simulink model "TosAeb"  (R2021a)
settings: Solver=FixedStepDiscrete  StartTime=0.0  StopTime=inf  FixedStep=0.01  RelTol=1e-3
undefined symbols (not defined in the file or in its folder): ACCModSts, AccSts1, AEB_ObjectMovingOrientation, AEB_ObjectMovingState, CN_BTCActive, CN_CurrentGearR, CN_CurrentGearR_DHT, CN_PTCActive, CN_VDCActive, CN_VSC_EspOff, CN_VSC_FCW_AEB_Error, CN_VSC_FCW_AEB_NoDispay, CN_VSC_FCW_AEB_PerfmDgdtn, CN_VSC_FCW_AEB_TempUnAvlbl, CN_VSC_VehStandstill, CNaccelAvoidMax, CNaccelAvoidMin, CNbrakePedalPress, CNcomfortStrLeftAccelMax, CNcomfortStrLeftAccelMin, CNcomfortStrRightAccelMax, CNcomfortStrRightAccelMin, CNDrvDoorOpen, CNDrvModeEco, CNDrvModeNorm, CNDrvModeOther, CNDrvModeSport, CNDrvSBRreminder, CNNull, CNobjInvalidID, CNPassDoorOpen, CNPassSBRreminder, CNPEBSAccelNegInf, CNPEBSAccelNull, CNPEBSTimeInf, CNPEBSTimeNull, CNSTOPMAX, CNSTOPNULL, CNsubjWidth, CNSwtOFF, CNSwtON, CNTTCMAX, CNTTCNULL, CNVehConfigAEBCycNone, CNVehConfigAEBNone, CNVehConfigAEBPedNone, CNVehConfigFCWNone, CNVehConfigJANone, CollisionSeverity, FALSE, FCWSnvty, JACycObjCalcInfoDefaultParams, JAPedObjCalcInfoDefaultParams, JAVehObjCalcInfoDefaultParams, latCycObjCalcInfoDefaultParams, latPedObjCalcInfoDefaultParams, longObjCalcInfoDefaultParams, ModSts, movingStatus, ObjectType ... (+1112)
libraries: simulink x3031, AEBSFunctionLib x50, Ta_Library x33, AEBSStateMachineLib x23, Mylibrary x12, simulink_extras x7, ObjSelect_lib x3, dspstat3 x2
(part 52 of 102)

== system TosAeb/FcwAebJa_Step_sys/ALG_AEBSystem/ALG_AEBController/AEB_FuncCondCalcApp/JaPedFunctionCondCalc/AEBJAPedPrefillSuppAborCond/AEBJAPedPrefillSuppCondCalc/JaPedPrefillSuppSubjCondCalcu/brakeReleventSuppCond ==
  VehConfigIbooster : Inport
  BrakePedalStatus_G : Inport  Port=2
  ESP_MasterCylindBrakePress : Inport  Port=3
  IB_BrkPedalAppld : Inport  Port=4
  Compare To Constant : simulink/Logic and Bit Operations/Compare To Constant  relop===  const=FALSE  OutDataTypeStr=boolean
  If : If  IfExpression=u1
  Merge : Merge
  brakeReleventSuppCond_ESP : Subsystem  VariantControl=(default)
  brakeReleventSuppCond_IBooster : Subsystem  VariantControl=P_AEB_PowerTrainConfig == 1
  brakeReleventSuppCond : Outport
  wires:
  BrakePedalStatus_G/1 -> brakeReleventSuppCond_ESP/1(BrakePedalStatus_G)
  ESP_MasterCylindBrakePress/1 -> brakeReleventSuppCond_ESP/2(ESP_MasterCylindBrakePress)
  IB_BrkPedalAppld/1 -> brakeReleventSuppCond_IBooster/1(IB_BrkPedalAppld)
  Merge/1 -> brakeReleventSuppCond/1
  brakeReleventSuppCond_IBooster/1(brakeReleventSuppCond) -> Merge/2
  brakeReleventSuppCond_ESP/1(brakeReleventSuppCond) -> Merge/1
  If/2 -> brakeReleventSuppCond_IBooster/Action
  If/1 -> brakeReleventSuppCond_ESP/Action
  Compare To Constant/1 -> If/1
  VehConfigIbooster/1 -> Compare To Constant/1

== system TosAeb/FcwAebJa_Step_sys/ALG_AEBSystem/ALG_AEBController/AEB_FuncCondCalcApp/JaVehFunctionCondCalc/AEBJAVehAEBfullSuppAborCond/AEBJAVehAEBfullSuppCondCalc/AEBJAVehAEBfullSuppSubjCondCalcu/brakeReleventSuppCond ==
  VehConfigIbooster : Inport
  ESP_MasterCylindBrakePress : Inport  Port=2
  Compare To Constant : simulink/Logic and Bit Operations/Compare To Constant  relop===  const=FALSE  OutDataTypeStr=boolean
  If : If  IfExpression=u1
  Merge : Merge
  brakeReleventSuppCond_ESP : Subsystem  VariantControl=(default)
  brakeReleventSuppCond_IBooster : Subsystem  VariantControl=P_AEB_PowerTrainConfig == 1
  brakeReleventSuppCond : Outport
  wires:
  ESP_MasterCylindBrakePress/1 -> brakeReleventSuppCond_ESP/1(ESP_MasterCylindBrakePress)
  Merge/1 -> brakeReleventSuppCond/1
  brakeReleventSuppCond_IBooster/1(brakeReleventSuppCond) -> Merge/2
  brakeReleventSuppCond_ESP/1(brakeReleventSuppCond) -> Merge/1
  If/2 -> brakeReleventSuppCond_IBooster/Action
  If/1 -> brakeReleventSuppCond_ESP/Action
  Compare To Constant/1 -> If/1
  VehConfigIbooster/1 -> Compare To Constant/1

== system TosAeb/FcwAebJa_Step_sys/ALG_AEBSystem/ALG_AEBController/AEB_FuncCondCalcApp/JaVehFunctionCondCalc/AEBJAVehPrefillSuppAborCond/AEBJAVehPrefillSuppCondCalc/AEBJAVehPrefillSuppSubjCondCalcu/brakeReleventSuppCond ==
  VehConfigIbooster : Inport
  BrakePedalStatus_G : Inport  Port=2
  ESP_MasterCylindBrakePress : Inport  Port=3
  IB_BrkPedalAppld : Inport  Port=4
  Compare To Constant : simulink/Logic and Bit Operations/Compare To Constant  relop===  const=FALSE  OutDataTypeStr=boolean
  If : If  IfExpression=u1
  Merge : Merge
  brakeReleventSuppCond_ESP : Subsystem  VariantControl=(default)
  brakeReleventSuppCond_IBooster : Subsystem  VariantControl=P_AEB_PowerTrainConfig == 1
  brakeReleventSuppCond : Outport
  wires:
  ESP_MasterCylindBrakePress/1 -> brakeReleventSuppCond_ESP/2(ESP_MasterCylindBrakePress)
  BrakePedalStatus_G/1 -> brakeReleventSuppCond_ESP/1(BrakePedalStatus_G)
  IB_BrkPedalAppld/1 -> brakeReleventSuppCond_IBooster/1(IB_BrkPedalAppld)
  Merge/1 -> brakeReleventSuppCond/1
  brakeReleventSuppCond_IBooster/1(brakeReleventSuppCond) -> Merge/2
  brakeReleventSuppCond_ESP/1(brakeReleventSuppCond) -> Merge/1
  If/2 -> brakeReleventSuppCond_IBooster/Action
  If/1 -> brakeReleventSuppCond_ESP/Action
  Compare To Constant/1 -> If/1
  VehConfigIbooster/1 -> Compare To Constant/1

== system TosAeb/FcwAebJa_Step_sys/ALG_AEBSystem/ALG_AEBController/AEB_FuncCondCalcApp/LongFunctionCondCalc/vehABASuppAborCondCalc/vehABAAborCondCalc/vehABAVehRelAborCondCalc/DriverAborCondJudge ==
  SteerWheelAngle : Inport
  SteerWheelSpd : Inport  Port=2
  AccrPedlPosnDiagc : Inport  Port=3
  Abs : Abs
  Abs1 : Abs
  Compare To Constant : simulink/Logic and Bit Operations/Compare To Constant  relop=>  const=P_AEB_Func_vehABAStrWheelAngleAborThres  OutDataTypeStr=boolean
  Compare To Constant1 : simulink/Logic and Bit Operations/Compare To Constant  relop=>  const=P_AEB_Func_vehABAStrWheelAngleSpdAborThres  OutDataTypeStr=boolean
  Compare To Constant4 : simulink/Logic and Bit Operations/Compare To Constant  relop=>  const=P_AEB_Func_vehABAGasPedalAborThres  OutDataTypeStr=boolean
  Logical Operator : Logic  Operator=OR  AllPortsSameDT=off  OutDataTypeStr=boolean
  Logical Operator2 : Logic  Operator=OR  AllPortsSameDT=off  OutDataTypeStr=boolean
  driverAborCondFlg : Outport
  wires:
  Logical Operator2/1 -> driverAborCondFlg/1  "driverAborCond"
  Compare To Constant4/1 -> Logical Operator2/2  "accrlReleventAborCond"
  AccrPedlPosnDiagc/1 -> Compare To Constant4/1
  Logical Operator/1 -> Logical Operator2/1  "strReleventAborCond"
  Compare To Constant1/1 -> Logical Operator/2
  Compare To Constant/1 -> Logical Operator/1
  Abs1/1 -> Compare To Constant1/1
  SteerWheelSpd/1 -> Abs1/1
  Abs/1 -> Compare To Constant/1
  SteerWheelAngle/1 -> Abs/1

== system TosAeb/FcwAebJa_Step_sys/ALG_AEBSystem/ALG_AEBController/AEB_FuncCondCalcApp/LongFunctionCondCalc/vehABASuppAborCondCalc/vehABAAborCondCalc/vehABAVehRelAborCondCalc/vehPreWarningVehRelActiveCondJudge ==
  driverAborCondFlg : Inport
  vehStateCalcABAAborFlg : Inport  Port=2
  Compare To Constant : simulink/Logic and Bit Operations/Compare To Constant  relop===  const=TRUE  OutDataTypeStr=boolean
  Compare To Constant1 : simulink/Logic and Bit Operations/Compare To Constant  relop===  const=TRUE  OutDataTypeStr=boolean
  Logical Operator1 : Logic  Operator=OR  AllPortsSameDT=off  OutDataTypeStr=boolean
  vehABAVehRelAborCondFulfilled : Outport
  wires:
  Logical Operator1/1 -> vehABAVehRelAborCondFulfilled/1
  vehStateCalcABAAborFlg/1 -> Compare To Constant1/1
  driverAborCondFlg/1 -> Compare To Constant/1
  Compare To Constant/1 -> Logical Operator1/1
  Compare To Constant1/1 -> Logical Operator1/2

== system TosAeb/FcwAebJa_Step_sys/ALG_AEBSystem/ALG_AEBController/AEB_FuncCondCalcApp/LongFunctionCondCalc/vehABASuppAborCondCalc/vehABASuppCondCalc/vehABAVehRelActiveCondCalc/DriverCondJudge ==
  SteerWheelAngle : Inport
  SteerWheelSpd : Inport  Port=2
  AccrPedlPosnDiagc : Inport  Port=3
  Abs : Abs
  Abs1 : Abs
  AccrlReleventSuppCondStateDelayAbortion : AEBSFunctionLib/StateDelayAbortion  SystemSampleTime=-1  VariantActivationTime=update diagram  Latency=0  AutoFrameSizeCalculation=off
  Compare To Constant : simulink/Logic and Bit Operations/Compare To Constant  relop=>  const=P_AEB_Func_vehABAStrWheelAngleActivationThres  OutDataTypeStr=boolean
  Compare To Constant1 : simulink/Logic and Bit Operations/Compare To Constant  relop=>  const=P_AEB_Func_vehABAStrWheelAngleSpdActivationThres  OutDataTypeStr=boolean
  Compare To Constant4 : simulink/Logic and Bit Operations/Compare To Constant  relop=>  const=P_AEB_Func_vehABAGasPedalActivationThres  OutDataTypeStr=boolean
  Constant : Constant  Value=P_AEB_Func_vehABAStrCondAborDelayTime
  Constant1 : Constant  Value=Ts
  Constant2 : Constant  Value=P_AEB_Func_vehABAAccrCondAborDelayTime
  Constant3 : Constant  Value=Ts
  Logical Operator : Logic  Operator=OR  AllPortsSameDT=off  OutDataTypeStr=boolean
  Logical Operator2 : Logic  AllPortsSameDT=off  OutDataTypeStr=boolean
  StrReleventSuppCondStateDelayAbortion : AEBSFunctionLib/StateDelayAbortion  SystemSampleTime=-1  VariantActivationTime=update diagram  Latency=0  AutoFrameSizeCalculation=off
  driverSuppCondFlg : Outport
  wires:
  Constant3/1 -> AccrlReleventSuppCondStateDelayAbortion/3
  Constant2/1 -> AccrlReleventSuppCondStateDelayAbortion/2
  Compare To Constant4/1 -> AccrlReleventSuppCondStateDelayAbortion/1
  Constant1/1 -> StrReleventSuppCondStateDelayAbortion/3
  Constant/1 -> StrReleventSuppCondStateDelayAbortion/2
  Logical Operator/1 -> StrReleventSuppCondStateDelayAbortion/1
  SteerWheelAngle/1 -> Abs/1
  Abs/1 -> Compare To Constant/1
  SteerWheelSpd/1 -> Abs1/1
  Abs1/1 -> Compare To Constant1/1
  Compare To Constant/1 -> Logical Operator/1
  Compare To Constant1/1 -> Logical Operator/2
  StrReleventSuppCondStateDelayAbortion/1 -> Logical Operator2/1  "strReleventSuppCond"
  AccrPedlPosnDiagc/1 -> Compare To Constant4/1
  AccrlReleventSuppCondStateDelayAbortion/1 -> Logical Operator2/2  "accrlReleventSuppCond"
  Logical Operator2/1 -> driverSuppCondFlg/1  "driverSuppCond"

== system TosAeb/FcwAebJa_Step_sys/ALG_AEBSystem/ALG_AEBController/AEB_FuncCondCalcApp/LongFunctionCondCalc/vehABASuppAborCondCalc/vehABASuppCondCalc/vehABAVehRelActiveCondCalc/subjVelWork ==
  ObjectMovingState : Inport
  Compare To Constant : simulink/Logic and Bit Operations/Compare To Constant  relop===  const=AEB_ObjectMovingState.Still  OutDataTypeStr=boolean
  Constant : Constant  Value=P_AEB_Func_vehABASubjVelMaxSO
  Constant2 : Constant  Value=P_AEB_Func_vehABASubjVelMin
  Constant3 : Constant  Value=P_AEB_Func_vehABASubjVelMaxMO
  Switch1 : Switch  Criteria=u2 ~= 0
  subjVelWorkMax : Outport
  subjVelWorkMin : Outport  Port=2
  wires:
  Switch1/1 -> subjVelWorkMax/1
  Constant3/1 -> Switch1/3
  Compare To Constant/1 -> Switch1/2
  Constant2/1 -> subjVelWorkMin/1
  ObjectMovingState/1 -> Compare To Constant/1
  Constant/1 -> Switch1/1

== system TosAeb/FcwAebJa_Step_sys/ALG_AEBSystem/ALG_AEBController/AEB_FuncCondCalcApp/LongFunctionCondCalc/vehABASuppAborCondCalc/vehABASuppCondCalc/vehABAVehRelActiveCondCalc/vehABAVehRelActiveCondJudge ==
  subjVel : Inport
  subjVelWorkMax : Inport  Port=2
  subjVelWorkMin : Inport  Port=3
  driverSuppCondFlg : Inport  Port=4
  vehStateCalcABASuppFlg : Inport  Port=5
  Compare To Constant : simulink/Logic and Bit Operations/Compare To Constant  relop===  const=FALSE  OutDataTypeStr=boolean
  Compare To Constant1 : simulink/Logic and Bit Operations/Compare To Constant  relop===  const=FALSE  OutDataTypeStr=boolean
  Logical Operator : Logic  AllPortsSameDT=off  OutDataTypeStr=boolean
  Logical Operator1 : Logic  Inputs=3  AllPortsSameDT=off  OutDataTypeStr=boolean
  Relational Operator : RelationalOperator  Operator=<  OutDataTypeStr=boolean  RndMeth=Simplest
  Relational Operator1 : RelationalOperator  Operator=>  OutDataTypeStr=boolean  RndMeth=Simplest
  vehABAVehRelActiveCondFulfilled : Outport
  wires:
  Compare To Constant1/1 -> Logical Operator1/3
  subjVel/1 -> Relational Operator1/1, Relational Operator/1
  subjVelWorkMax/1 -> Relational Operator/2
  Relational Operator/1 -> Logical Operator/1
  Relational Operator1/1 -> Logical Operator/2
  driverSuppCondFlg/1 -> Compare To Constant/1
  vehStateCalcABASuppFlg/1 -> Compare To Constant1/1
  Compare To Constant/1 -> Logical Operator1/2
  Logical Operator/1 -> Logical Operator1/1
  Logical Operator1/1 -> vehABAVehRelActiveCondFulfilled/1
  subjVelWorkMin/1 -> Relational Operator1/2

== system TosAeb/FcwAebJa_Step_sys/ALG_AEBSystem/ALG_AEBController/AEB_FuncCondCalcApp/LongFunctionCondCalc/vehAEBfullSuppAborCondCalc/ vehAEBfullSuppCondCalc/vehAEBfullSituationRelActiveCondCalc/overlapThesCalc ==
  ObjectTypeCameraConfirmed : Inport
  dxFrontBumper : Inport  Port=2
  Compare To Constant : simulink/Logic and Bit Operations/Compare To Constant  relop===  const=TRUE  OutDataTypeStr=boolean
  If : If  IfExpression=u1
  If Action Subsystem : Subsystem  TreatAsAtomicUnit=on
  If Action Subsystem1 : Subsystem  TreatAsAtomicUnit=on
  Merge : Merge
  overlapThes : Outport
  wires:
  ObjectTypeCameraConfirmed/1 -> Compare To Constant/1
  dxFrontBumper/1 -> If Action Subsystem1/1(dxFrontBumper), If Action Subsystem/1(dxFrontBumper)
  If Action Subsystem/1(overlapThes) -> Merge/1
  Compare To Constant/1 -> If/1
  [ObjectNoFusion] -> If Action Subsystem1/Action
  Merge/1 -> overlapThes/1
  If/2 -> [ObjectNoFusion]
  If Action Subsystem1/1(overlapThes) -> Merge/2
  If/1 -> If Action Subsystem/Action

== system TosAeb/FcwAebJa_Step_sys/ALG_AEBSystem/ALG_AEBController/AEB_FuncCondCalcApp/LongFunctionCondCalc/vehAEBfullSuppAborCondCalc/ vehAEBfullSuppCondCalc/vehAEBfullSituationRelActiveCondCalc/vehAEBfullSituationRelActiveCondCalc ==
  overlapThes : Inport
  longCollisionSeverity : Inport  Port=2
  overlapRatio : Inport  Port=3
  LCK_Active : Inport  Port=4
  probEscape : Inport  Port=5
  subjCurvTraj : Inport  Port=6
  Compare To Constant1 : simulink/Logic and Bit Operations/Compare To Constant  relop===  const=TRUE  OutDataTypeStr=boolean
  Compare To Constant2 : simulink/Logic and Bit Operations/Compare To Constant  relop===  const=CollisionSeverity.High  OutDataTypeStr=boolean
  Compare To Constant3 : simulink/Logic and Bit Operations/Compare To Constant  relop=>  const=P_AEB_Func_vehAEBfullEscapeActivationThres  OutDataTypeStr=boolean
  Compare To Constant4 : simulink/Logic and Bit Operations/Compare To Constant  relop=<  const=P_AEB_Func_vehAEBfullSubjCurvTrajActivationThres  OutDataTypeStr=boolean
  Compare To Constant5 : simulink/Logic and Bit Operations/Compare To Constant  relop===  const=FALSE  OutDataTypeStr=boolean
  Constant : Constant  Value=TRUE
  Logical Operator1 : Logic  Inputs=4  AllPortsSameDT=off  OutDataTypeStr=boolean
  Relational Operator : RelationalOperator  Operator=>  OutDataTypeStr=boolean  RndMeth=Simplest
  Switch : Switch  Criteria=u2 ~= 0
  vehAEBfullSituationRelActiveCondFulfilled : Outport
  wires:
  Constant/1 -> Switch/1
  Switch/1 -> Logical Operator1/3
  Compare To Constant1/1 -> Switch/2
  LCK_Active/1 -> Compare To Constant1/1
  longCollisionSeverity/1 -> Compare To Constant2/1
  subjCurvTraj/1 -> Compare To Constant4/1
  overlapThes/1 -> Relational Operator/2
  overlapRatio/1 -> Relational Operator/1
  probEscape/1 -> Compare To Constant3/1
  Compare To Constant3/1 -> Compare To Constant5/1  "probEscapeFulfilled"
  Compare To Constant5/1 -> Switch/3
  Relational Operator/1 -> Logical Operator1/2  "OverlapCondFulfilled"
  Compare To Constant4/1 -> Logical Operator1/4  "latStableCondFulfilled"
  Compare To Constant2/1 -> Logical Operator1/1  "collisionLvlCondFulfilled"
  Logical Operator1/1 -> vehAEBfullSituationRelActiveCondFulfilled/1

== system TosAeb/FcwAebJa_Step_sys/ALG_AEBSystem/ALG_AEBController/AEB_FuncCondCalcApp/LongFunctionCondCalc/vehAEBfullSuppAborCondCalc/ vehAEBfullSuppCondCalc/vehAEBfullVehRelActiveCondCalc/DriverCondJudge ==
  SteerWheelAngle : Inport
  SteerWheelSpd : Inport  Port=2
  VehConfigIbooster : Inport  Port=3
  ESPmasterCylindBrakePress : Inport  Port=4
  BrakePedalStatus_G : Inport  Port=5
  subjLongAccel : Inport  Port=6
  subjVel : Inport  Port=7
  AccrPedlPosnDiagc : Inport  Port=8
  Abs : Abs
  Abs1 : Abs
  Compare To Constant : simulink/Logic and Bit Operations/Compare To Constant  relop=>  const=P_AEB_Func_vehAEBfullStrWheelAngleActivationThres  OutDataTypeStr=boolean
  Compare To Constant1 : simulink/Logic and Bit Operations/Compare To Constant  relop=>  const=P_AEB_Func_vehAEBfullStrWheelAngleSpdActivationThres  OutDataTypeStr=boolean
  Compare To Constant4 : simulink/Logic and Bit Operations/Compare To Constant  relop=>  const=P_AEB_Func_vehAEBfullGasPedalActivationThres  OutDataTypeStr=boolean
  Constant : Constant  Value=P_AEB_Func_vehAEBfullStrCondAborDelayTime
  Constant1 : Constant  Value=Ts
  Constant2 : Constant  Value=P_AEB_Func_vehAEBfullAccrCondAborDelayTime
  Constant3 : Constant  Value=Ts
  Logical Operator : Logic  Operator=OR  AllPortsSameDT=off  OutDataTypeStr=boolean
  Logical Operator2 : Logic  Operator=OR  Inputs=3  AllPortsSameDT=off  OutDataTypeStr=boolean
  accrlReleventSuppCondStateDelayAbortion : AEBSFunctionLib/StateDelayAbortion  SystemSampleTime=-1  VariantActivationTime=update diagram  Latency=0  AutoFrameSizeCalculation=off
  brakeReleventSuppCond : Subsystem
  strReleventSuppCondStateDelayAbortion : AEBSFunctionLib/StateDelayAbortion  SystemSampleTime=-1  VariantActivationTime=update diagram  Latency=0  AutoFrameSizeCalculation=off
  driverSuppCondFlg : Outport
  wires:
  VehConfigIbooster/1 -> brakeReleventSuppCond/1(VehConfigIbooster)
  ESPmasterCylindBrakePress/1 -> brakeReleventSuppCond/2(ESPmasterCylindBrakePress)
  subjVel/1 -> brakeReleventSuppCond/5(subjVel)
  BrakePedalStatus_G/1 -> brakeReleventSuppCond/3(BrakePedalStatus_G)
  subjLongAccel/1 -> brakeReleventSuppCond/4(subjLongAccel)
  Constant3/1 -> accrlReleventSuppCondStateDelayAbortion/3
  Constant2/1 -> accrlReleventSuppCondStateDelayAbortion/2
  Compare To Constant4/1 -> accrlReleventSuppCondStateDelayAbortion/1
  Constant1/1 -> strReleventSuppCondStateDelayAbortion/3
  Constant/1 -> strReleventSuppCondStateDelayAbortion/2
  SteerWheelAngle/1 -> Abs/1
  Abs/1 -> Compare To Constant/1
  SteerWheelSpd/1 -> Abs1/1
  Abs1/1 -> Compare To Constant1/1
  Compare To Constant/1 -> Logical Operator/1
  Compare To Constant1/1 -> Logical Operator/2
  Logical Operator/1 -> strReleventSuppCondStateDelayAbortion/1
  strReleventSuppCondStateDelayAbortion/1 -> Logical Operator2/1  "strReleventSuppCond"
  brakeReleventSuppCond/1(brakeReleventSuppCond) -> Logical Operator2/2  "brakeReleventSuppCond"
  AccrPedlPosnDiagc/1 -> Compare To Constant4/1
  accrlReleventSuppCondStateDelayAbortion/1 -> Logical Operator2/3  "accrlReleventSuppCond"
  Logical Operator2/1 -> driverSuppCondFlg/1  "driverSuppCond"

== system TosAeb/FcwAebJa_Step_sys/ALG_AEBSystem/ALG_AEBController/AEB_FuncCondCalcApp/LongFunctionCondCalc/vehAEBfullSuppAborCondCalc/ vehAEBfullSuppCondCalc/vehAEBfullVehRelActiveCondCalc/subjVelWork ==
  ObjectTypeCameraConfirmed : Inport
  ObjectMovingState : Inport  Port=2
  Compare To Constant : simulink/Logic and Bit Operations/Compare To Constant  relop===  const=AEB_ObjectMovingState.Still  OutDataTypeStr=boolean
  Compare To Constant1 : simulink/Logic and Bit Operations/Compare To Constant  relop===  const=TRUE  OutDataTypeStr=boolean
  Constant : Constant  Value=P_AEB_Func_vehAEBfullFusionSubjVelMaxThresSO
  Constant1 : Constant  Value=P_AEB_Func_vehAEBfullNoFusionSubjVelMaxThresSO
  Constant2 : Constant  Value=P_AEB_Func_vehAEBfullSubjVelMinThres
  Constant3 : Constant  Value=P_AEB_Func_vehAEBfullSubjWorkVelMaxThresMO
  Switch : Switch  Criteria=u2 ~= 0
  Switch1 : Switch  Criteria=u2 ~= 0
  subjVelWorkMax : Outport
  subjVelWorkMin : Outport  Port=2
  wires:
  Switch1/1 -> subjVelWorkMax/1
  Constant3/1 -> Switch1/3
  Switch/1 -> Switch1/1
  Compare To Constant/1 -> Switch1/2
  Compare To Constant1/1 -> Switch/2
  ObjectTypeCameraConfirmed/1 -> Compare To Constant1/1
  Constant2/1 -> subjVelWorkMin/1
  Constant1/1 -> Switch/3
  Constant/1 -> Switch/1
  ObjectMovingState/1 -> Compare To Constant/1

== system TosAeb/FcwAebJa_Step_sys/ALG_AEBSystem/ALG_AEBController/AEB_FuncCondCalcApp/LongFunctionCondCalc/vehAEBfullSuppAborCondCalc/ vehAEBfullSuppCondCalc/vehAEBfullVehRelActiveCondCalc/vehAEBfullVehRelActiveCondJudge ==
  subjVel : Inport
  subjVelWorkMax : Inport  Port=2
  subjVelWorkMin : Inport  Port=3
  driverSuppCondFlg : Inport  Port=4
  vehStateCalcAEBfullSuppFlg : Inport  Port=5
  Compare To Constant : simulink/Logic and Bit Operations/Compare To Constant  relop===  const=FALSE  OutDataTypeStr=boolean
  Compare To Constant1 : simulink/Logic and Bit Operations/Compare To Constant  relop===  const=FALSE  OutDataTypeStr=boolean
  Logical Operator : Logic  AllPortsSameDT=off  OutDataTypeStr=boolean
  Logical Operator1 : Logic  Inputs=3  AllPortsSameDT=off  OutDataTypeStr=boolean
  Relational Operator : RelationalOperator  Operator=<  OutDataTypeStr=boolean  RndMeth=Simplest
  Relational Operator1 : RelationalOperator  Operator=>  OutDataTypeStr=boolean  RndMeth=Simplest
  vehAEBfullVehRelActiveCondFulfilled : Outport
  wires:
  Compare To Constant1/1 -> Logical Operator1/3
  subjVel/1 -> Relational Operator1/1, Relational Operator/1
  subjVelWorkMax/1 -> Relational Operator/2
  Relational Operator/1 -> Logical Operator/1
  Relational Operator1/1 -> Logical Operator/2
  driverSuppCondFlg/1 -> Compare To Constant/1
  vehStateCalcAEBfullSuppFlg/1 -> Compare To Constant1/1
  Compare To Constant/1 -> Logical Operator1/2
  Logical Operator/1 -> Logical Operator1/1
  Logical Operator1/1 -> vehAEBfullVehRelActiveCondFulfilled/1  "vehAEBfullVehRelActiveCondFulfilled"
  subjVelWorkMin/1 -> Relational Operator1/2

== system TosAeb/FcwAebJa_Step_sys/ALG_AEBSystem/ALG_AEBController/AEB_FuncCondCalcApp/LongFunctionCondCalc/vehAEBfullSuppAborCondCalc/vehAEBfullAborCondCalc/vehAEBfullVehRelAborCondCalc/DriverAborCondJudge ==
  SteerWheelAngle : Inport
  SteerWheelSpd : Inport  Port=2
  AccrPedlPosnDiagc : Inport  Port=3
  Abs : Abs
  Abs1 : Abs
  Compare To Constant : simulink/Logic and Bit Operations/Compare To Constant  relop=>  const=P_AEB_Func_vehAEBfullStrWheelAngleAborThres  OutDataTypeStr=boolean
  Compare To Constant1 : simulink/Logic and Bit Operations/Compare To Constant  relop=>  const=P_AEB_Func_vehAEBfullStrWheelAngleSpdAborThres  OutDataTypeStr=boolean
  Compare To Constant4 : simulink/Logic and Bit Operations/Compare To Constant  relop=>  const=P_AEB_Func_vehAEBfullGasPedalAborThres  OutDataTypeStr=boolean
  Logical Operator : Logic  Operator=OR  AllPortsSameDT=off  OutDataTypeStr=boolean
  Logical Operator2 : Logic  Operator=OR  AllPortsSameDT=off  OutDataTypeStr=boolean
  driverAborCondFlg : Outport
  wires:
  Logical Operator2/1 -> driverAborCondFlg/1  "driverAborCond"
  Compare To Constant4/1 -> Logical Operator2/2  "accrlReleventAborCond"
  AccrPedlPosnDiagc/1 -> Compare To Constant4/1
  Logical Operator/1 -> Logical Operator2/1  "strReleventAborCond"
  Compare To Constant1/1 -> Logical Operator/2
  Compare To Constant/1 -> Logical Operator/1
  Abs1/1 -> Compare To Constant1/1
  SteerWheelSpd/1 -> Abs1/1
  Abs/1 -> Compare To Constant/1
  SteerWheelAngle/1 -> Abs/1

== system TosAeb/FcwAebJa_Step_sys/ALG_AEBSystem/ALG_AEBController/AEB_FuncCondCalcApp/LongFunctionCondCalc/vehAEBfullSuppAborCondCalc/vehAEBfullAborCondCalc/vehAEBfullVehRelAborCondCalc/vehAEBfullVehRelAborCondJudge ==
  driverAborCondFlg : Inport
  vehStateCalcAEBfullAborFlg : Inport  Port=2
  Compare To Constant : simulink/Logic and Bit Operations/Compare To Constant  relop===  const=TRUE  OutDataTypeStr=boolean
  Compare To Constant1 : simulink/Logic and Bit Operations/Compare To Constant  relop===  const=TRUE  OutDataTypeStr=boolean
  Logical Operator1 : Logic  Operator=OR  AllPortsSameDT=off  OutDataTypeStr=boolean
  vehAEBfullVehRelAborCondFulfilled : Outport
  wires:
  Compare To Constant1/1 -> Logical Operator1/2
  Logical Operator1/1 -> vehAEBfullVehRelAborCondFulfilled/1  "vehAEBfullVehRelAborCondFulfilled"
  Compare To Constant/1 -> Logical Operator1/1
  vehStateCalcAEBfullAborFlg/1 -> Compare To Constant1/1  "vehStateCalcAEBfullAborFlg"
  driverAborCondFlg/1 -> Compare To Constant/1

== system TosAeb/FcwAebJa_Step_sys/ALG_AEBSystem/ALG_AEBController/AEB_FuncCondCalcApp/LongFunctionCondCalc/vehAEBpartSuppAborCondCalc/vehAEBpartAborCondCalc/vehAEBpartVehRelAborCondCalc/DriverAborCondJudge ==
  SteerWheelAngle : Inport
  SteerWheelSpd : Inport  Port=2
  AccrPedlPosnDiagc : Inport  Port=3
  Abs : Abs
  Abs1 : Abs
  Compare To Constant : simulink/Logic and Bit Operations/Compare To Constant  relop=>  const=P_AEB_Func_vehAEBpartStrWheelAngleSpdAborThres  OutDataTypeStr=boolean
  Compare To Constant1 : simulink/Logic and Bit Operations/Compare To Constant  relop=>  const=P_AEB_Func_vehAEBpartStrWheelAngleAborThres  OutDataTypeStr=boolean
  Compare To Constant4 : simulink/Logic and Bit Operations/Compare To Constant  relop=>  const=P_AEB_Func_vehAEBpartGasPedalAborThres  OutDataTypeStr=boolean
  Logical Operator : Logic  Operator=OR  AllPortsSameDT=off  OutDataTypeStr=boolean
  Logical Operator2 : Logic  Operator=OR  AllPortsSameDT=off  OutDataTypeStr=boolean
  driverAborCondFlg : Outport
  wires:
  SteerWheelAngle/1 -> Abs/1
  Abs/1 -> Compare To Constant/1
  SteerWheelSpd/1 -> Abs1/1
  Abs1/1 -> Compare To Constant1/1
  Compare To Constant/1 -> Logical Operator/1
  Compare To Constant1/1 -> Logical Operator/2
  Logical Operator/1 -> Logical Operator2/1  "strReleventAborCond"
  AccrPedlPosnDiagc/1 -> Compare To Constant4/1
  Compare To Constant4/1 -> Logical Operator2/2  "accrlReleventAborCond"
  Logical Operator2/1 -> driverAborCondFlg/1  "driverAborCondFlg"

== system TosAeb/FcwAebJa_Step_sys/ALG_AEBSystem/ALG_AEBController/AEB_FuncCondCalcApp/LongFunctionCondCalc/vehAEBpartSuppAborCondCalc/vehAEBpartAborCondCalc/vehAEBpartVehRelAborCondCalc/vehPreWarningVehRelActiveCondJudge ==
  note: driverAborCondFlg
  note: vehStateCalcAEBpartAborFlg
  driverAborCondFlg : Inport
  vehStateCalcAEBpartAborFlg : Inport  Port=2
  Compare To Constant : simulink/Logic and Bit Operations/Compare To Constant  relop===  const=TRUE  OutDataTypeStr=boolean
  Compare To Constant1 : simulink/Logic and Bit Operations/Compare To Constant  relop===  const=TRUE  OutDataTypeStr=boolean
  Logical Operator1 : Logic  Operator=OR  AllPortsSameDT=off  OutDataTypeStr=boolean
  vehAEBpartVehRelAborCondFulfilled : Outport
  wires:
  driverAborCondFlg/1 -> Compare To Constant/1
  vehStateCalcAEBpartAborFlg/1 -> Compare To Constant1/1
  Compare To Constant/1 -> Logical Operator1/1
  Logical Operator1/1 -> vehAEBpartVehRelAborCondFulfilled/1  "vehAEBpartVehRelAborCondFulfilled"
  Compare To Constant1/1 -> Logical Operator1/2

== system TosAeb/FcwAebJa_Step_sys/ALG_AEBSystem/ALG_AEBController/AEB_FuncCondCalcApp/LongFunctionCondCalc/vehAEBpartSuppAborCondCalc/vehAEBpartSuppCondCalc/vehAEBpartObjRelActiveCondCalc/AEBpartVruFuncConfig ==
  ObjectType : Inport
  Compare To Constant : simulink/Logic and Bit Operations/Compare To Constant  relop===  const=ObjectTypeCopy.BICYCLE  OutDataTypeStr=boolean
  Compare To Constant1 : simulink/Logic and Bit Operations/Compare To Constant  relop===  const=FALSE  OutDataTypeStr=boolean
  Compare To Constant11 : simulink/Logic and Bit Operations/Compare To Constant  relop===  const=ObjectTypeCopy.PEDESTRIAN  OutDataTypeStr=boolean
  Constant : Constant  Value=P_AEB_Func_vehAEBpartVruFuncConfig
  Constant1 : Constant  Value=TRUE
  Logical Operator3 : Logic  Operator=OR  AllPortsSameDT=off  OutDataTypeStr=boolean
  Switch : Switch  Criteria=u2 ~= 0
  vehAEBpartVruFuncConfig : Outport
  wires:
  Constant1/1 -> Switch/1
  Compare To Constant1/1 -> Switch/3
  Constant/1 -> Switch/2
  Switch/1 -> vehAEBpartVruFuncConfig/1
  Logical Operator3/1 -> Compare To Constant1/1
  ObjectType/1 -> Compare To Constant/1, Compare To Constant11/1
  Compare To Constant/1 -> Logical Operator3/1
  Compare To Constant11/1 -> Logical Operator3/2

== system TosAeb/FcwAebJa_Step_sys/ALG_AEBSystem/ALG_AEBController/AEB_FuncCondCalcApp/LongFunctionCondCalc/vehAEBpartSuppAborCondCalc/vehAEBpartSuppCondCalc/vehAEBpartSituationRelActiveCondCalc/overlapThesCalc ==
  ObjectTypeCameraConfirmed : Inport
  dxFrontBumper : Inport  Port=2
  Compare To Constant : simulink/Logic and Bit Operations/Compare To Constant  relop===  const=TRUE  OutDataTypeStr=boolean
  If : If  IfExpression=u1
  If Action Subsystem : Subsystem  TreatAsAtomicUnit=on
  If Action Subsystem1 : Subsystem  TreatAsAtomicUnit=on
  Merge : Merge
  overlapThes : Outport
  wires:
  ObjectTypeCameraConfirmed/1 -> Compare To Constant/1
  dxFrontBumper/1 -> If Action Subsystem1/1(dxFrontBumper), If Action Subsystem/1(dxFrontBumper)
  If Action Subsystem/1(overlapThes) -> Merge/1
  Compare To Constant/1 -> If/1
  [ObjectNoFusion] -> If Action Subsystem1/Action
  Merge/1 -> overlapThes/1
  If/2 -> [ObjectNoFusion]
  If Action Subsystem1/1(overlapThes) -> Merge/2
  If/1 -> If Action Subsystem/Action

== system TosAeb/FcwAebJa_Step_sys/ALG_AEBSystem/ALG_AEBController/AEB_FuncCondCalcApp/LongFunctionCondCalc/vehAEBpartSuppAborCondCalc/vehAEBpartSuppCondCalc/vehAEBpartSituationRelActiveCondCalc/vehAEBpartSituationRelActiveCondCalc ==
  longCollisionSeverity : Inport
  overlapRatio : Inport  Port=2
  OverlapThes : Inport  Port=3
  LCK_Active : Inport  Port=4
  probEscape : Inport  Port=5
  subjCurvTraj : Inport  Port=6
  Compare To Constant1 : simulink/Logic and Bit Operations/Compare To Constant  relop===  const=CollisionSeverity.Medium  OutDataTypeStr=boolean
  Compare To Constant2 : simulink/Logic and Bit Operations/Compare To Constant  relop===  const=CollisionSeverity.High  OutDataTypeStr=boolean
  Compare To Constant3 : simulink/Logic and Bit Operations/Compare To Constant  relop=>  const=P_AEB_Func_vehAEBpartEscapeActivationThres  OutDataTypeStr=boolean
  Compare To Constant4 : simulink/Logic and Bit Operations/Compare To Constant  relop=<  const=P_AEB_Func_vehAEBpartSubjCurvTrajActivationThres  OutDataTypeStr=boolean
  Compare To Constant5 : simulink/Logic and Bit Operations/Compare To Constant  relop===  const=FALSE  OutDataTypeStr=boolean
  Compare To Constant7 : simulink/Logic and Bit Operations/Compare To Constant  relop===  const=TRUE  OutDataTypeStr=boolean
  Constant : Constant  Value=TRUE
  Logical Operator : Logic  Operator=OR  AllPortsSameDT=off  OutDataTypeStr=boolean
  Logical Operator1 : Logic  Inputs=4  AllPortsSameDT=off  OutDataTypeStr=boolean
  Relational Operator : RelationalOperator  Operator=>  OutDataTypeStr=boolean  RndMeth=Simplest
  Switch : Switch  Criteria=u2 ~= 0
  vehAEBpartSituationRelActiveCondFulfilled : Outport
  wires:
  Switch/1 -> Logical Operator1/3
  Compare To Constant5/1 -> Switch/3
  Constant/1 -> Switch/1
  LCK_Active/1 -> Compare To Constant7/1
  Compare To Constant7/1 -> Switch/2
  OverlapThes/1 -> Relational Operator/2
  Logical Operator1/1 -> vehAEBpartSituationRelActiveCondFulfilled/1  "vehAEBpartSituationRelActiveCondFulfilled"
  Logical Operator/1 -> Logical Operator1/1  "collisionLvlCondFulfilled"
  Compare To Constant4/1 -> Logical Operator1/4  "latStableCondFulfilled"
  Relational Operator/1 -> Logical Operator1/2  "OverlapCondFulfilled"
  Compare To Constant3/1 -> Compare To Constant5/1  "probEscapeFulfilled"
  subjCurvTraj/1 -> Compare To Constant4/1
  probEscape/1 -> Compare To Constant3/1
  overlapRatio/1 -> Relational Operator/1
  Compare To Constant2/1 -> Logical Operator/2
  Compare To Constant1/1 -> Logical Operator/1
  longCollisionSeverity/1 -> Compare To Constant2/1, Compare To Constant1/1

== system TosAeb/FcwAebJa_Step_sys/ALG_AEBSystem/ALG_AEBController/AEB_FuncCondCalcApp/LongFunctionCondCalc/vehAEBpartSuppAborCondCalc/vehAEBpartSuppCondCalc/vehAEBpartVehRelActiveCondCalc/DriverCondJudge ==
  SteerWheelAngle : Inport
  SteerWheelSpd : Inport  Port=2
  AccrPedlPosnDiagc : Inport  Port=3
  VehConfigIbooster : Inport  Port=4
  ESPmasterCylindBrakePress : Inport  Port=5
  BrakePedalStatus_G : Inport  Port=6
  IB_BrkPedalAppld : Inport  Port=7
  Abs : Abs
  Abs1 : Abs
  Compare To Constant : simulink/Logic and Bit Operations/Compare To Constant  relop=>  const=P_AEB_Func_vehAEBpartStrWheelAngleActivationThres  OutDataTypeStr=boolean
  Compare To Constant1 : simulink/Logic and Bit Operations/Compare To Constant  relop=>  const=P_AEB_Func_vehAEBpartStrWheelAngleSpdActivationThres  OutDataTypeStr=boolean
  Compare To Constant4 : simulink/Logic and Bit Operations/Compare To Constant  relop=>  const=P_AEB_Func_vehAEBpartGasPedalActivationThres  OutDataTypeStr=boolean
  Constant : Constant  Value=P_AEB_Func_vehAEBpartStrCondAborDelayTime
  Constant1 : Constant  Value=Ts
  Constant2 : Constant  Value=P_AEB_Func_vehAEBpartAccrCondAborDelayTime
  Constant3 : Constant  Value=Ts
  Logical Operator : Logic  Operator=OR  AllPortsSameDT=off  OutDataTypeStr=boolean
  Logical Operator2 : Logic  Operator=OR  Inputs=3  AllPortsSameDT=off  OutDataTypeStr=boolean
  StateDelayAbortion1 : AEBSFunctionLib/StateDelayAbortion  SystemSampleTime=-1  VariantActivationTime=update diagram  Latency=0  AutoFrameSizeCalculation=off
  StateDelayAbortion2 : AEBSFunctionLib/StateDelayAbortion  SystemSampleTime=-1  VariantActivationTime=update diagram  Latency=0  AutoFrameSizeCalculation=off
  brakeReleventSuppCond : Subsystem
  driverSuppCondFlg : Outport
  wires:
  VehConfigIbooster/1 -> brakeReleventSuppCond/1(VehConfigIbooster)
  ESPmasterCylindBrakePress/1 -> brakeReleventSuppCond/2(ESPmasterCylindBrakePress)
  BrakePedalStatus_G/1 -> brakeReleventSuppCond/3(BrakePedalStatus_G)
  IB_BrkPedalAppld/1 -> brakeReleventSuppCond/4(IB_BrkPedalAppld)
  Compare To Constant4/1 -> StateDelayAbortion2/1
  Constant3/1 -> StateDelayAbortion2/3
  Constant2/1 -> StateDelayAbortion2/2
  Constant1/1 -> StateDelayAbortion1/3
  Constant/1 -> StateDelayAbortion1/2
  Logical Operator/1 -> StateDelayAbortion1/1
  Logical Operator2/1 -> driverSuppCondFlg/1  "driverSuppCondFlg"
  StateDelayAbortion2/1 -> Logical Operator2/3  "accrlReleventSuppCond"
  AccrPedlPosnDiagc/1 -> Compare To Constant4/1
  brakeReleventSuppCond/1(brakeReleventSuppCond) -> Logical Operator2/2  "brakeReleventSuppCond"
  StateDelayAbortion1/1 -> Logical Operator2/1  "strReleventSuppCond"
  Compare To Constant1/1 -> Logical Operator/2
  Compare To Constant/1 -> Logical Operator/1
  Abs1/1 -> Compare To Constant1/1
  SteerWheelSpd/1 -> Abs1/1
  Abs/1 -> Compare To Constant/1
  SteerWheelAngle/1 -> Abs/1

== system TosAeb/FcwAebJa_Step_sys/ALG_AEBSystem/ALG_AEBController/AEB_FuncCondCalcApp/LongFunctionCondCalc/vehAEBpartSuppAborCondCalc/vehAEBpartSuppCondCalc/vehAEBpartVehRelActiveCondCalc/vehAEBpartVehRelActiveCondJudge ==
  subjVel : Inport
  driverSuppCondFlg : Inport  Port=2
  vehStateCalcAEBpartSuppFlg : Inport  Port=3
  Compare To Constant : simulink/Logic and Bit Operations/Compare To Constant  relop===  const=FALSE  OutDataTypeStr=boolean
  Compare To Constant1 : simulink/Logic and Bit Operations/Compare To Constant  relop===  const=FALSE  OutDataTypeStr=boolean
  Constant1 : Constant  Value=P_AEB_Func_vehAEBpartSubjVelMaxActivationThres
  Constant2 : Constant  Value=P_AEB_Func_vehAEBpartSubjVelMinActivationThres
  Logical Operator : Logic  AllPortsSameDT=off  OutDataTypeStr=boolean
  Logical Operator1 : Logic  Inputs=3  AllPortsSameDT=off  OutDataTypeStr=boolean
  Relational Operator : RelationalOperator  Operator=<  OutDataTypeStr=boolean  RndMeth=Simplest
  Relational Operator1 : RelationalOperator  Operator=>  OutDataTypeStr=boolean  RndMeth=Simplest
  vehAEBpartVehRelActiveCondFulfilled : Outport
  wires:
  Constant2/1 -> Relational Operator1/2
  Logical Operator1/1 -> vehAEBpartVehRelActiveCondFulfilled/1  "vehAEBpartVehRelActiveCondFulfilled"
  Logical Operator/1 -> Logical Operator1/1
  Compare To Constant/1 -> Logical Operator1/2
  vehStateCalcAEBpartSuppFlg/1 -> Compare To Constant1/1  "vehStateCalcAEBpartSuppFlg"
  driverSuppCondFlg/1 -> Compare To Constant/1  "driverSuppCondFlg"
  Relational Operator1/1 -> Logical Operator/2
  Relational Operator/1 -> Logical Operator/1
  Constant1/1 -> Relational Operator/2
  subjVel/1 -> Relational Operator1/1, Relational Operator/1
  Compare To Constant1/1 -> Logical Operator1/3

== system TosAeb/FcwAebJa_Step_sys/ALG_AEBSystem/ALG_AEBController/AEB_FuncCondCalcApp/LongFunctionCondCalc/vehAcuteWarningSuppAborCondFulfilled/vehAcuteWarningAborCondCalc/vehAcuteWarningVehRelAborCondCalc/DriverAborCondJudge ==
  SteerWheelAngle : Inport
  SteerWheelSpd : Inport  Port=2
  AccrPedlPosnDiagc : Inport  Port=3
  Abs : Abs
  Abs1 : Abs
  Compare To Constant : simulink/Logic and Bit Operations/Compare To Constant  relop=>  const=P_AEB_Func_vehAcuteWarningStrWheelAngleAborThres  OutDataTypeStr=boolean
  Compare To Constant1 : simulink/Logic and Bit Operations/Compare To Constant  relop=>  const=P_AEB_Func_vehAcuteWarningStrWheelAngleSpdAborThres  OutDataTypeStr=boolean
  Compare To Constant4 : simulink/Logic and Bit Operations/Compare To Constant  relop=>  const=P_AEB_Func_vehAcuteWarningGasPedalAborThres  OutDataTypeStr=boolean
  Logical Operator : Logic  Operator=OR  AllPortsSameDT=off  OutDataTypeStr=boolean
  Logical Operator2 : Logic  Operator=OR  AllPortsSameDT=off  OutDataTypeStr=boolean
  driverAborCondFlg : Outport
  wires:
  Logical Operator2/1 -> driverAborCondFlg/1  "driverAborCondFlg"
  Compare To Constant4/1 -> Logical Operator2/2  "accrlReleventAborCond"
  AccrPedlPosnDiagc/1 -> Compare To Constant4/1
  Logical Operator/1 -> Logical Operator2/1  "strReleventAborCond"
  Compare To Constant1/1 -> Logical Operator/2
  Compare To Constant/1 -> Logical Operator/1
  Abs1/1 -> Compare To Constant1/1
  SteerWheelSpd/1 -> Abs1/1
  Abs/1 -> Compare To Constant/1
  SteerWheelAngle/1 -> Abs/1

== system TosAeb/FcwAebJa_Step_sys/ALG_AEBSystem/ALG_AEBController/AEB_FuncCondCalcApp/LongFunctionCondCalc/vehAcuteWarningSuppAborCondFulfilled/vehAcuteWarningAborCondCalc/vehAcuteWarningVehRelAborCondCalc/vehPreWarningVehRelActiveCondJudge ==
  driverAborCondFlg : Inport
  vehStateCalcAcuteWarningAborFlg : Inport  Port=2
  Compare To Constant : simulink/Logic and Bit Operations/Compare To Constant  relop===  const=TRUE  OutDataTypeStr=boolean
  Compare To Constant1 : simulink/Logic and Bit Operations/Compare To Constant  relop===  const=TRUE  OutDataTypeStr=boolean
  Logical Operator1 : Logic  Operator=OR  AllPortsSameDT=off  OutDataTypeStr=boolean
  vehAcuteWarningVehRelAborCondFulfilled : Outport
  wires:
  Compare To Constant1/1 -> Logical Operator1/2
  Logical Operator1/1 -> vehAcuteWarningVehRelAborCondFulfilled/1  "vehAcuteWarningVehRelAborCondFulfilled"
  Compare To Constant/1 -> Logical Operator1/1
  vehStateCalcAcuteWarningAborFlg/1 -> Compare To Constant1/1  "vehStateCalcAcuteWarningAborFlg"
  driverAborCondFlg/1 -> Compare To Constant/1  "driverAborCondFlg"

== system TosAeb/FcwAebJa_Step_sys/ALG_AEBSystem/ALG_AEBController/AEB_FuncCondCalcApp/LongFunctionCondCalc/vehAcuteWarningSuppAborCondFulfilled/vehAcuteWarningSuppCondCalc/vehAcuteWarningVehRelActiveCondCalc/BrakeNeedCondJudge ==
  BrakePedalStatus_G : Inport
  subjLongAccel : Inport  Port=2
  Compare To Constant3 : simulink/Logic and Bit Operations/Compare To Constant  relop===  const=CNbrakePedalPress  OutDataTypeStr=boolean
  Compare To Constant5 : simulink/Logic and Bit Operations/Compare To Constant  relop=<  const=P_AEB_Func_vehAcuteWarningSubjLongAccelActivationThres  OutDataTypeStr=boolean
  Logical Operator1 : Logic  Operator=NOT  AllPortsSameDT=off  OutDataTypeStr=boolean
  Logical Operator3 : Logic  AllPortsSameDT=off  OutDataTypeStr=boolean
  brakeNeedCondFulfilled : Outport
  wires:
  Logical Operator1/1 -> brakeNeedCondFulfilled/1  "brakeNeedCondFulfilled"
  Compare To Constant3/1 -> Logical Operator3/1
  Logical Operator3/1 -> Logical Operator1/1
  subjLongAccel/1 -> Compare To Constant5/1
  Compare To Constant5/1 -> Logical Operator3/2
  BrakePedalStatus_G/1 -> Compare To Constant3/1

== system TosAeb/FcwAebJa_Step_sys/ALG_AEBSystem/ALG_AEBController/AEB_FuncCondCalcApp/LongFunctionCondCalc/vehAcuteWarningSuppAborCondFulfilled/vehAcuteWarningSuppCondCalc/vehAcuteWarningVehRelActiveCondCalc/DriverCondJudge ==
  SteerWheelAngle : Inport
  SteerWheelSpd : Inport  Port=2
  VehConfigIbooster : Inport  Port=3
  AccrPedlPosnDiagc : Inport  Port=4
  ESPmasterCylindBrakePress : Inport  Port=5
  IB_BrkPedalAppld : Inport  Port=6
  BrakePedalStatus_G : Inport  Port=7
  Abs : Abs
  Abs1 : Abs
  Compare To Constant : simulink/Logic and Bit Operations/Compare To Constant  relop=>  const=P_AEB_Func_vehAcuteWarningStrWheelAngleActivationThres  OutDataTypeStr=boolean
  Compare To Constant1 : simulink/Logic and Bit Operations/Compare To Constant  relop=>  const=P_AEB_Func_vehAcuteWarningStrWheelAngleSpdActivationThres  OutDataTypeStr=boolean
  Compare To Constant4 : simulink/Logic and Bit Operations/Compare To Constant  relop=>  const=P_AEB_Func_vehAcuteWarningGasPedalActivationThres  OutDataTypeStr=boolean
  Constant : Constant  Value=P_AEB_Func_vehAcuteWarningStrCondAborDelayTime
  Constant1 : Constant  Value=Ts
  Constant2 : Constant  Value=P_AEB_Func_vehAcuteWarningAccrCondAborDelayTime
  Constant3 : Constant  Value=Ts
  Logical Operator : Logic  Operator=OR  AllPortsSameDT=off  OutDataTypeStr=boolean
  Logical Operator2 : Logic  Inputs=3  AllPortsSameDT=off  OutDataTypeStr=boolean
  StateDelayAbortion : AEBSFunctionLib/StateDelayAbortion  SystemSampleTime=-1  VariantActivationTime=update diagram  Latency=0  AutoFrameSizeCalculation=off
  accrlReleventSuppCondStateDelayAbortion : AEBSFunctionLib/StateDelayAbortion  SystemSampleTime=-1  VariantActivationTime=update diagram  Latency=0  AutoFrameSizeCalculation=off
  brakeReleventSuppCond : Subsystem
  driverSuppCondFlg : Outport
  wires:
  VehConfigIbooster/1 -> brakeReleventSuppCond/1(VehConfigIbooster)
  IB_BrkPedalAppld/1 -> brakeReleventSuppCond/4(IB_BrkPedalAppld)
  BrakePedalStatus_G/1 -> brakeReleventSuppCond/3(BrakePedalStatus_G)
  ESPmasterCylindBrakePress/1 -> brakeReleventSuppCond/2(ESPmasterCylindBrakePress)
  Constant3/1 -> accrlReleventSuppCondStateDelayAbortion/3
  Constant2/1 -> accrlReleventSuppCondStateDelayAbortion/2
  Constant1/1 -> StateDelayAbortion/3
  Constant/1 -> StateDelayAbortion/2
  Logical Operator/1 -> StateDelayAbortion/1  "strReleventSuppCond"
  SteerWheelAngle/1 -> Abs/1
  Abs/1 -> Compare To Constant/1
  SteerWheelSpd/1 -> Abs1/1
  Abs1/1 -> Compare To Constant1/1
  Compare To Constant/1 -> Logical Operator/1
  Compare To Constant1/1 -> Logical Operator/2
  StateDelayAbortion/1 -> Logical Operator2/1  "strReleventSuppCond"
  brakeReleventSuppCond/1(brakeReleventSuppCond) -> Logical Operator2/2  "brakeReleventSuppCond"
  AccrPedlPosnDiagc/1 -> Compare To Constant4/1
  accrlReleventSuppCondStateDelayAbortion/1 -> Logical Operator2/3  "accrlReleventSuppCond"
  Compare To Constant4/1 -> accrlReleventSuppCondStateDelayAbortion/1
  Logical Operator2/1 -> driverSuppCondFlg/1  "driverSuppCondFlg"

== system TosAeb/FcwAebJa_Step_sys/ALG_AEBSystem/ALG_AEBController/AEB_FuncCondCalcApp/LongFunctionCondCalc/vehAcuteWarningSuppAborCondFulfilled/vehAcuteWarningSuppCondCalc/vehAcuteWarningVehRelActiveCondCalc/subjVelWork ==
  ObjectMovingState : Inport
  Compare To Constant : simulink/Logic and Bit Operations/Compare To Constant  relop===  const=AEB_ObjectMovingState.Still  OutDataTypeStr=boolean
  Constant : Constant  Value=P_AEB_Func_vehAcuteWarningSubjVelMaxActivationThresSO
  Constant1 : Constant  Value=P_AEB_Func_vehAcuteWarningSubjVelMaxActivationThresMO
  Constant2 : Constant  Value=P_AEB_Func_vehAcuteWarningSubjVelMinActivationThres
  Switch : Switch  Criteria=u2 ~= 0
  subjVelWorkMax : Outport
  subjVelWorkMin : Outport  Port=2
  wires:
  Constant2/1 -> subjVelWorkMin/1
  Switch/1 -> subjVelWorkMax/1
  Constant1/1 -> Switch/3
  Constant/1 -> Switch/1
  ObjectMovingState/1 -> Compare To Constant/1
  Compare To Constant/1 -> Switch/2

== system TosAeb/FcwAebJa_Step_sys/ALG_AEBSystem/ALG_AEBController/AEB_FuncCondCalcApp/LongFunctionCondCalc/vehAcuteWarningSuppAborCondFulfilled/vehAcuteWarningSuppCondCalc/vehAcuteWarningVehRelActiveCondCalc/vehAcuteWarningVehRelActiveCondJudge ==
  subjVel : Inport
  subjVelWorkMax : Inport  Port=2
  subjVelWorkMin : Inport  Port=3
  driverSuppCondFlg : Inport  Port=4
  brakeNeedCondFulfilled : Inport  Port=5
  vehStateCalcPreWarningSuppFlg : Inport  Port=6
  Compare To Constant : simulink/Logic and Bit Operations/Compare To Constant  relop===  const=FALSE  OutDataTypeStr=boolean
  Compare To Constant1 : simulink/Logic and Bit Operations/Compare To Constant  relop===  const=FALSE  OutDataTypeStr=boolean
  Compare To Constant2 : simulink/Logic and Bit Operations/Compare To Constant  relop===  const=TRUE  OutDataTypeStr=boolean
  Logical Operator : Logic  AllPortsSameDT=off  OutDataTypeStr=boolean
  Logical Operator1 : Logic  Inputs=4  AllPortsSameDT=off  OutDataTypeStr=boolean
  Relational Operator : RelationalOperator  Operator=<  OutDataTypeStr=boolean  RndMeth=Simplest
  Relational Operator1 : RelationalOperator  Operator=>  OutDataTypeStr=boolean  RndMeth=Simplest
  vehAcuteWarningVehRelActiveCondFulfilled : Outport
  wires:
  Compare To Constant1/1 -> Logical Operator1/4
  Compare To Constant2/1 -> Logical Operator1/3
  brakeNeedCondFulfilled/1 -> Compare To Constant2/1
  subjVel/1 -> Relational Operator1/1, Relational Operator/1
  subjVelWorkMax/1 -> Relational Operator/2
  subjVelWorkMin/1 -> Relational Operator1/2
  Relational Operator/1 -> Logical Operator/1
  Relational Operator1/1 -> Logical Operator/2
  driverSuppCondFlg/1 -> Compare To Constant/1
  vehStateCalcPreWarningSuppFlg/1 -> Compare To Constant1/1
  Compare To Constant/1 -> Logical Operator1/2
  Logical Operator/1 -> Logical Operator1/1
  Logical Operator1/1 -> vehAcuteWarningVehRelActiveCondFulfilled/1  "vehAcuteWarningVehRelActiveCondFulfilled"

== system TosAeb/FcwAebJa_Step_sys/ALG_AEBSystem/ALG_AEBController/AEB_FuncCondCalcApp/LongFunctionCondCalc/vehEBASuppAborCondCalc/vehEBAAborCondCalc/vehEBAVehRelAborCondCalc/BrkAborCondJudge ==
  BrakePedalStatus_G : Inport
  ESPmasterCylindBrakePress : Inport  Port=2
  Compare To Constant2 : simulink/Logic and Bit Operations/Compare To Constant  relop=~=  const=CNbrakePedalPress  OutDataTypeStr=boolean
  Compare To Constant3 : simulink/Logic and Bit Operations/Compare To Constant  relop=<  const=P_AEB_Func_vehEBSMasterCylindBrkPressAborThres  OutDataTypeStr=boolean
  Logical Operator1 : Logic  Operator=OR  AllPortsSameDT=off  OutDataTypeStr=boolean
  BrkAborCondFlg : Outport
  wires:
  Compare To Constant3/1 -> Logical Operator1/2
  Compare To Constant2/1 -> Logical Operator1/1
  ESPmasterCylindBrakePress/1 -> Compare To Constant3/1
  BrakePedalStatus_G/1 -> Compare To Constant2/1
  Logical Operator1/1 -> BrkAborCondFlg/1

== system TosAeb/FcwAebJa_Step_sys/ALG_AEBSystem/ALG_AEBController/AEB_FuncCondCalcApp/LongFunctionCondCalc/vehEBASuppAborCondCalc/vehEBAAborCondCalc/vehEBAVehRelAborCondCalc/DriverAborCondJudge ==
  SteerWheelAngle : Inport
  SteerWheelSpd : Inport  Port=2
  AccrPedlPosnDiagc : Inport  Port=3
  Abs : Abs
  Abs1 : Abs
  Compare To Constant : simulink/Logic and Bit Operations/Compare To Constant  relop=>  const=P_AEB_Func_vehEBAGasPedalAborThres  OutDataTypeStr=boolean
  Compare To Constant1 : simulink/Logic and Bit Operations/Compare To Constant  relop=>  const=P_AEB_Func_vehEBAStrWheelAngleSpdAborThres  OutDataTypeStr=boolean
  Compare To Constant4 : simulink/Logic and Bit Operations/Compare To Constant  relop=>  const=P_AEB_Func_vehEBAStrWheelAngleAborThres  OutDataTypeStr=boolean
  Logical Operator : Logic  Operator=OR  AllPortsSameDT=off  OutDataTypeStr=boolean
  Logical Operator2 : Logic  Operator=OR  AllPortsSameDT=off  OutDataTypeStr=boolean
  driverAborCondFlg : Outport
  wires:
  SteerWheelAngle/1 -> Abs/1
  Abs/1 -> Compare To Constant/1
  SteerWheelSpd/1 -> Abs1/1
  Abs1/1 -> Compare To Constant1/1
  Compare To Constant/1 -> Logical Operator/1
  Compare To Constant1/1 -> Logical Operator/2
  Logical Operator/1 -> Logical Operator2/1  "strReleventAborCond"
  AccrPedlPosnDiagc/1 -> Compare To Constant4/1
  Compare To Constant4/1 -> Logical Operator2/2  "accrlReleventAborCond"
  Logical Operator2/1 -> driverAborCondFlg/1  "driverAborCond"

== system TosAeb/FcwAebJa_Step_sys/ALG_AEBSystem/ALG_AEBController/AEB_FuncCondCalcApp/LongFunctionCondCalc/vehEBASuppAborCondCalc/vehEBAAborCondCalc/vehEBAVehRelAborCondCalc/vehPreWarningVehRelActiveCondJudge ==
  BrkAborCondFlg : Inport
  driverAborCondFlg : Inport  Port=2
  vehStateCalcEBAAborFlg : Inport  Port=3
  Compare To Constant : simulink/Logic and Bit Operations/Compare To Constant  relop===  const=TRUE  OutDataTypeStr=boolean
  Compare To Constant1 : simulink/Logic and Bit Operations/Compare To Constant  relop===  const=TRUE  OutDataTypeStr=boolean
  Compare To Constant2 : simulink/Logic and Bit Operations/Compare To Constant  relop===  const=TRUE  OutDataTypeStr=boolean
  Logical Operator1 : Logic  Operator=OR  Inputs=3  AllPortsSameDT=off  OutDataTypeStr=boolean
  vehEBAVehRelAborCondFulfilled : Outport
  wires:
  Compare To Constant2/1 -> Logical Operator1/1
  Compare To Constant1/1 -> Logical Operator1/3
  Compare To Constant/1 -> Logical Operator1/2
  BrkAborCondFlg/1 -> Compare To Constant2/1
  driverAborCondFlg/1 -> Compare To Constant/1
  vehStateCalcEBAAborFlg/1 -> Compare To Constant1/1
  Logical Operator1/1 -> vehEBAVehRelAborCondFulfilled/1
